simulink library "ee_lib_for_power_systems"  (R2025b)
settings: Solver=VariableStepAuto  StartTime=0.0  StopTime=10.0  RelTol=1e-3
undefined symbols (not defined in the file or in its folder): Alt_PolePair_Count, C3m, DiscIntNeverNeededParam, Enum1to12, L3m, Total_SM_Inertia, Ts
libraries: fl_lib x291, nesl_utility x219, ee_lib x139, custom_lib x12, $bdroot x10, ee_sl_lib x3, eeTransforms x2, eeGeneralControl x1, simulink x1
(part 2 of 11)

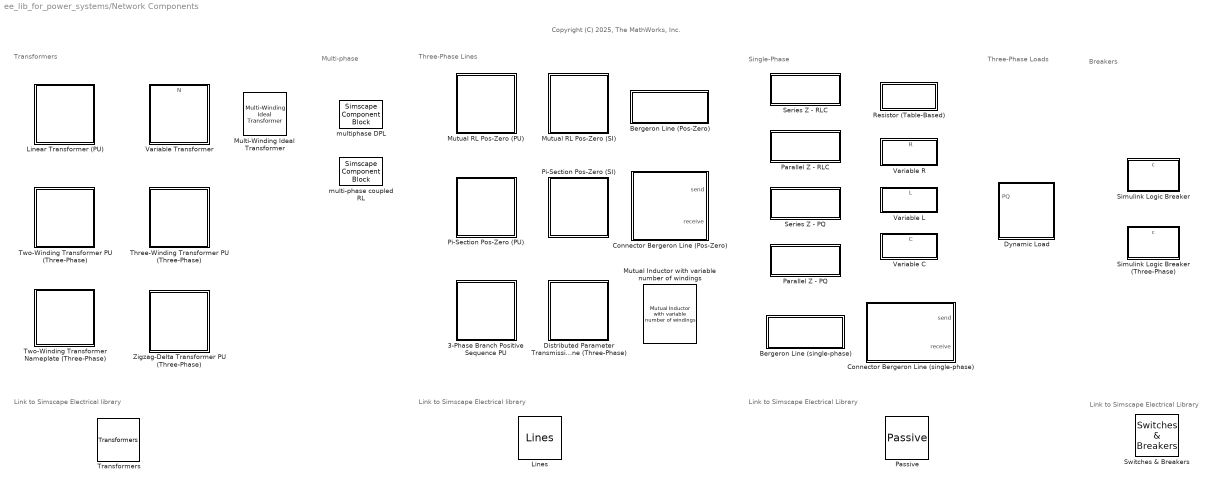
== system ee_lib_for_power_systems/Network Components ==
  note: Multi-phase
  note: Link to Simscape Electrical Library
  note: Link to Simscape Electrical Library
  note: Link to Simscape Electrical library
  note: Link to Simscape Electrical library
  note: Three-Phase Loads
  note: Breakers
  note: Single-Phase
  note: Three-Phase Lines
  note: Transformers
  note: Copyright (C) 2025, The MathWorks, Inc.
  " Three-Winding Transformer PU (Three-Phase)" : Subsystem  mask{conn1=Yg, conn2=delta1, conn3=Y, core=fivelimb, Pnom_Fnom=[250e6 60], V1_R1_L1=[300000 0.01 0.05], V2_R2_L2=[50000 0.01 0.05], V3_R3_L3=[100000 0.01 0.05], Rm_Lm=[500 500], run_time=on}
      mask prompts: conn1 "Winding 1 Connection"; conn2 "Winding 2 Connection"; conn3 "Winding 3 Connection"; Pnom_Fnom "Nominal values [Pnom(VA) Fnom(Hz)]"; V1_R1_L1 "Winding 1 [V1(Vrms) R1(pu) L1(pu)]"; V2_R2_L2 "Winding 2 [V2(Vrms) R2(pu) L2(pu)]"; V3_R3_L3 "Winding 3 [V3(Vrms) R3(pu) L3(pu)]"; Rm_Lm "Magnetization [Rm (pu) Lm(pu)]"; run_time "Set parameters to run-time (model does not recompile if parameters change value)"
      mask init:
      | maskObj = Simulink.Mask.get(gcbh);
      | workspaceObj = maskObj.getWorkspace();
      | 
      | Pnom_Fnom = workspaceObj.get('Pnom_Fnom');
      | V1_R1_L1 = workspaceObj.get('V1_R1_L1');
      | V2_R2_L2 = workspaceObj.get('V2_R2_L2');
      | V3_R3_L3 = workspaceObj.get('V3_R3_L3');
      | Rm_Lm = workspaceObj.get('Rm_Lm');
      | 
      | R1base = V1_R1_L1(1)^2/Pnom_Fnom(1);
      | X1base = V1_R1_L1(1)^2/Pnom_Fnom(1)/(2*pi*Pnom_Fnom(2));
      | 
      | R2base = V2_R2_L2(1)^2/Pnom_Fnom(1);
      | X2base = V2_R2_L2(1)^2/Pnom_Fnom(1)/(2*pi*Pnom_Fnom(2));
      | 
      | R3base = V3_R3_L3(1)^2/Pnom_Fnom(1);
      | X3base = V3_R3_L3(1)^2/Pnom_Fnom(1)/(2*pi*Pnom_Fnom(2));
      | 
      | V1 = V1_R1_L1(1);
      | V2 = V2_R2_L2(1);
      | V3 = V3_R3_L3(1);
      | 
      | R1_pu = V1_R1_L1(2);
      | L1_pu = V1_R1_L1(3);
      | R2_pu = V2_R2_L2(2);
      | L2_pu = V2_R2_L2(3);
      | R3_pu = V3_R3_L3(2);
      | L3_pu = V3_R3_L3(3);
      | Rm_pu = Rm_Lm(1);
      | Lm_pu = Rm_Lm(2);
      |     
      | path_Transformer = [gcb,'/Transformer'];
      | 
      | conn1 = get_param(gcb,'conn1');
      | conn2 = get_param(gcb,'conn2');
      | conn3 = get_param(gcb,'conn3');
      | core  = get_param(gcb,'core');
      | 
      | set_param(path_Transformer,'Winding1Connection',['ee.enum.windingconnection.',conn1])
      | set_param(path_Transformer,'Winding2Connection',['ee.enum.windingconnection.',conn2])
      | set_param(path_Transformer,'Winding3Connection',['ee.enum.windingconnection.',conn3])
      | set_param(path_Transformer,'CoreType',['ee.enum.coretype.',core])
      mask TypeOptions conn1: Y | Yg | delta1 | delta11
      mask TypeOptions conn2: Y | Yg | delta1 | delta11
      mask TypeOptions conn3: Y | Yg | delta1 | delta11
      mask TypeOptions core: threelimb | fivelimb
      mask Callback run_time:
      | run_time = get_param(gcb,'run_time');
      | 
      | path_Transformer = [gcb,'/Transformer'];
      | 
      | switch run_time
      |     case 'off'
      |         pcr = 'compiletime';
      |     case 'on'
      |         pcr = 'runtime';
      | end
      | 
      | set_param(path_Transformer,'VRated1_conf',pcr)
      | set_param(path_Transformer,'VRated2_conf',pcr)
      | set_param(path_Transformer,'VRated3_conf',pcr)
      | set_param(path_Transformer,'SRated_conf',pcr)
      | set_param(path_Transformer,'FRated_conf',pcr)
      | set_param(path_Transformer,'pu_Rm_conf',pcr)
      | set_param(path_Transformer,'pu_Xm_conf',pcr)
      | set_param(path_Transformer,'pu_Rw1_conf',pcr)
      | set_param(path_Transformer,'pu_Xl1_conf',pcr)
      | set_param(path_Transformer,'pu_Rw2_conf',pcr)
      | set_param(path_Transformer,'pu_Xl2_conf',pcr)
      | set_param(path_Transformer,'pu_Rw3_conf',pcr)
      | set_param(path_Transformer,'pu_Xl3_conf',pcr)
      mask DialogControl DescGroupVar Prompt: Three-Winding Transformer
      mask DialogControl Container4 Prompt: Configure
      mask DialogControl Container5 Prompt: Parameters
      mask Display:
      | color('lightblue')
      | 
      | ph = linspace(0,2*pi,200);
      | 
      | plot(0.2*sin(ph)+0.4,0.2*cos(ph)+0.5)
      | plot(0.2*sin(ph)+0.6,0.2*cos(ph)+0.62)
      | plot(0.2*sin(ph)+0.6,0.2*cos(ph)+0.38)
      | 
      | plot([0.1 0.2],[0.5 0.5])
      | plot([0.75 0.9],[0.25 0.25])
      | plot([0.75 0.9],[0.75 0.75])
      | plot(0,0)
      | plot(1,1)
      | 
      | conn1 = get_param(gcbh,'conn1');
      | cc1 = conn1;
      | if strcmp(conn1,'delta1')
      |     cc1 = 'D1';
      | elseif strcmp(conn1,'delta11')
      |     cc1 = 'D11';
      | end
      | 
      | text(0.2,0.25,cc1)
      | 
      | conn2 = get_param(gcbh,'conn2');
      | cc2 = conn2;
      | if strcmp(conn2,'delta1')
      |     cc2 = 'D1';
      | elseif strcmp(conn2,'delta11')
      |     cc2 = 'D11';
      | end
      | 
      | text(0.7,0.9,cc2)
      | 
      | conn3 = get_param(gcbh,'conn3');
      | cc3 = conn3;
      | if strcmp(conn3,'delta1')
      |     cc3 = 'D1';
      | elseif strcmp(conn3,'delta11')
      |     cc3 = 'D11';
      | end
      | 
      | text(0.7,0.15 ,cc3)
  3-Phase Branch Positive Sequence PU : Subsystem  mask{Pnom_Fnom=[5e6 60], Tx=off, Vnom1=5e3, Vnom2=2e3, R=0.1, X=0.1, B=0.1, run_time=on}
      mask prompts: Pnom_Fnom "Nominal values [Pnom(VA) Fnom(Hz)]"; Tx "Include Ideal Transformer"; Vnom1 "Port 1 Nominal Voltage (Vrms)"; Vnom2 "Nominal Voltage (Vrms)"; R "Series Resistance R (pu)"; X "Series Reactance X (pu)"; B "Shunt Line Charging Susceptance B (pu)"; run_time "Set parameters to run-time (avoid recompile if parameters change value)"
      mask init:
      | maskObj = Simulink.Mask.get(gcbh);
      | workspaceObj = maskObj.getWorkspace();
      | 
      | % Pnom_Vnom = workspaceObj.get('Pnom_Fnom');
      | % Vnom1 = workspaceObj.get('Vnom1');
      | % Vnom2 = workspaceObj.get('Vnom2');
      | 
      | R = workspaceObj.get('R');
      | X = workspaceObj.get('X');
      | B = workspaceObj.get('B');
      | 
      | path_RLC   = [gcb,'/RLC'];
      | path_C1    = [gcb,'/C1'];
      | path_C2    = [gcb,'/C2'];
      | path_gnd1  = [gcb,'/gnd1'];
      | path_gnd2  = [gcb,'/gnd2'];
      | 
      | 
      | if R>0 && X>0 % set branch to RL
      | 
      |     set_param(path_RLC,'component_structure','ee.enum.rlc.structure.SeriesRL');
      | 
      | elseif R>0 && X<0 % set branch to RC
      | 
      |     set_param(path_RLC,'component_structure','ee.enum.rlc.structure.SeriesRC');
      | 
      | elseif R==0 && X>0 % set branch to L
      | 
      |     set_param(path_RLC,'component_structure','ee.enum.rlc.structure.L');
      | 
      | elseif R==0 && X<0 % set branch to C
      | 
      |     set_param(path_RLC,'component_structure','ee.enum.rlc.structure.C');
      | 
      | end
      | 
      | if B>0 
      | 
      |     set_param(path_C1,'Commented','off')
      |     set_param(path_C2,'Commented','off')
      |     set_param(path_gnd1,'Commented','off')
      |     set_param(path_gnd2,'Commented','off')
      | 
      | elseif B==0 % comment out shunt
      | 
      |     set_param(path_C1,'Commented','on');
      |     set_param(path_C2,'Commented','on');
      |     set_param(path_gnd1,'Commented','on')
      |     set_param(path_gnd2,'Commented','on')
      |     
      | end
      mask Callback Tx:
      | Tx = get_param(gcb,'Tx');
      | 
      | path_Tx  = [gcb,'/Tx'];
      | 
      | mp  ={
      |     'Nominal values [Pnom(VA) Fnom(Hz)]'
      |     'Include Ideal Transformer'
      |     'Port 1 Nominal Voltage (Vrms)'
      |     'Nominal Voltage (Vrms)'
      |     'Series Resistance R (pu)'
      |     'Series Reactance X (pu)'
      |     'Shunt Line Charging Susceptance B (pu)'
      |     'Set parameters to run-time (avoid recompile if parameters change value)'
      |     };
      | 
      | set_param(gcb,'MaskPrompts',mp)
      | 
      | switch Tx
      | 
      |     case 'off'
      | 
      |         set_param(gcb,'MaskVisibilityString','on,on,off,on,on,on,on,on')
      |         set_param(path_Tx,'Commented','through')
      | 
      |     case 'on'
      | 
      |         set_param(gcb,'MaskVisibilityString','on,on,on,on,on,on,on,on')
      |         mp{4} = 'Port 2 Nominal Voltage (Vrms)';
      |         set_param(path_Tx,'Commented','off')
      | 
      | end
      | 
      | set_param(gcb,'MaskPrompts',mp)
      mask Callback run_time:
      | run_time = get_param(gcb,'run_time');
      | 
      | path_RLC   = [gcb,'/RLC'];
      | path_C1    = [gcb,'/C1'];
      | path_C2    = [gcb,'/C2'];
      | path_TtxA  = [gcb,'/Tx/TtxA'];
      | path_TtxB  = [gcb,'/Tx/TtxB'];
      | path_TtxC  = [gcb,'/Tx/TtxC'];
      | 
      | switch run_time
      |     case 'off'
      |         pcr = 'compiletime';
      |     case 'on'
      |         pcr = 'runtime';
      | end
      | 
      | set_param(path_RLC,'R_conf',pcr)
      | set_param(path_RLC,'L_conf',pcr)
      | set_param(path_RLC,'C_conf',pcr)
      | set_param(path_C1,'C_conf',pcr)
      | set_param(path_C2,'C_conf',pcr)
      | set_param(path_C1,'L_conf',pcr)
      | set_param(path_C2,'L_conf',pcr)
      | set_param(path_TtxA,'n_conf',pcr)
      | set_param(path_TtxB,'n_conf',pcr)
      | set_param(path_TtxC,'n_conf',pcr)
      mask DialogControl DescGroupVar Prompt: 3-Phase Branch Positive Sequence PU
      mask DialogControl DescTextVar Prompt: This branch is parameterized from positive sequence data in R, X, B format. Where R is series resistance, X is series reactance and B is line charging susceptance. For series inductance X is positive. For series capacitance, X is negative. When included, the ideal transformer is connected at port 1, and the RLC per-unit values are referred to the voltage at port 2.
      mask DialogControl Container5 Prompt: Parameters
      mask Display: disp('Three-Phase\nBranch\nPos-Seq (PU)')
  Bergeron Line (Pos-Zero) : Subsystem  mask{length=100, R1_L1_C1=[0.01 0.001 1e-8], R0_L0_C0=[0.4 0.004 8e-9], Ts=5e-5}
      mask prompts: length "Length (km)"; R1_L1_C1 "Positive sequence [R1 (Ohm/km)  L1 (H/km)  C1 (F/km)]"; R0_L0_C0 "Zero sequence [R0 (Ohm/km)  L0 (H/km)  C0 (F/km)]"; Ts "Sample time (s)"
      mask init:
      | maskObj = Simulink.Mask.get(gcb);
      | workspaceObj = maskObj.getWorkspace();
      | 
      | length   = workspaceObj.get('length');
      | R1_L1_C1 = workspaceObj.get('R1_L1_C1');
      | R0_L0_C0 = workspaceObj.get('R0_L0_C0');
      | 
      | R1 = R1_L1_C1(1);
      | L1 = R1_L1_C1(2);
      | C1 = R1_L1_C1(3);
      | 
      | R0 = R0_L0_C0(1);
      | L0 = R0_L0_C0(2);
      | C0 = R0_L0_C0(3);
      | 
      | 
      | Rp = (2*R1 + R0)/3;
      | Rm = (R0 - R1)/3;
      | 
      | Lp = (2*L1 + L0)/3;
      | Lm = (L0 - L1)/3;
      | 
      | Cp = (2*C1 + C0)/3;
      | Cm = (C0 - C1)/3;
      | 
      | 
      | % Clarke Transform for alpha-beta-zero to ABC
      | 
      | T_clarke = [ 2/sqrt(6)  0         1/sqrt(3)
      |             -1/sqrt(6)  1/sqrt(2) 1/sqrt(3)
      |             -1/sqrt(6) -1/sqrt(2) 1/sqrt(3)];
      | 
      | R_alpha = length*(Rp - Rm);
      | L_alpha = length*(Lp - Lm);
      | C_alpha = length*(Cp - Cm);
      | 
      | R_beta = length*(Rp - Rm);
      | L_beta = length*(Lp - Lm);
      | C_beta = length*(Cp - Cm);
      | 
      | R_zero = length*(Rp + 2*Rm);
      | L_zero = length*(Lp + 2*Lm);
      | C_zero = length*(Cp + 2*Cm);
      | 
      | Zlc_alpha = sqrt(L_alpha/C_alpha);
      | Zlc_beta  = sqrt(L_beta/C_beta);
      | Zlc_zero  = sqrt(L_zero/C_zero);
      | 
      | tau_alpha = sqrt(L_alpha*C_alpha);
      | tau_beta  = sqrt(L_beta*C_beta);
      | tau_zero  = sqrt(L_zero*C_zero);
      | 
      | h_alpha = (Zlc_alpha - R_alpha/4)/(Zlc_alpha + R_alpha/4);
      | h_beta  = (Zlc_beta - R_beta/4)/(Zlc_beta + R_beta/4);
      | h_zero  = (Zlc_zero - R_zero/4)/(Zlc_zero + R_zero/4);
      | 
      | Zc = T_clarke*diag([Zlc_alpha + R_alpha/4 Zlc_beta + R_beta/4 Zlc_zero + R_zero/4])*T_clarke';
      mask DialogControl DescGroupVar Prompt: Bergeron Line (Pos-Zero)
      mask DialogControl DescTextVar Prompt: Three-phase Bergeron line modeled in the Clarke domain and parameterized with positive- and zero-sequence values.
      mask Display: disp('Bergeron Line\n (Pos-Zero)')
  Bergeron Line (single-phase) : Subsystem  mask{length=100, freq=60, R1_L1_C1=[0.01 0.001 1e-8], Ts=5e-5}
      mask prompts: length "Length (km)"; freq "Frequency (Hz)"; R1_L1_C1 "[R (Ohm/km)  L (H/km)  C (F/km)]"; Ts "Sample time (s)"
      mask init:
      | maskObj = Simulink.Mask.get(gcb);
      | workspaceObj = maskObj.getWorkspace();
      | 
      | length   = workspaceObj.get('length');
      | R1_L1_C1 = workspaceObj.get('R1_L1_C1');
      | 
      | R1 = R1_L1_C1(1);
      | L1 = R1_L1_C1(2);
      | C1 = R1_L1_C1(3);
      | 
      | R = length*R1;
      | L = length*L1;
      | C = length*C1;
      | 
      | Zlc = sqrt(L/C);
      | 
      | tau = sqrt(L*C);
      | 
      | h = (Zlc - R/4)/(Zlc + R/4);
      | 
      | Zc = Zlc + R/4;
      mask DialogControl DescGroupVar Prompt: Bergeron Line (single-phase)
      mask DialogControl DescTextVar Prompt: Single-phase Bergeron line.
      mask Display: disp('Bergeron Line\n (single-phase)')
  Connector Bergeron Line (Pos-Zero) : Subsystem  mask{length=100, R1_L1_C1=[0.01 0.001 1e-8], R0_L0_C0=[0.4 0.004 8e-9], Ts=5e-5}
      mask prompts: length "Length (km)"; R1_L1_C1 "Positive sequence [R1 (Ohm/km) L1 (H/km) C1 (F/km)]"; R0_L0_C0 "Negative sequence [R0 (Ohm/km) L0 (H/km) C0 (F/km)]"; Ts "Sample time (s)"
      mask init: same as ee_lib_for_power_systems/Network Components/Bergeron Line (Pos-Zero)
      settings: PortSchema="{\"entries\":[{\"content\":{\"sides\":[{\"content\":{\"connectorIds\":[\"Out1\",\"In1\"]},\"type\":\"ConnectorPlacement.EquallySpacedRectSide\",\"uuid\":\"9f5ca3d6-f6a4-41cb-8fcc-925cc537c09c\"},{\"content\":{\"connectorIds\":[\"RConn1\"],\"side\":\"RIGHT\"},\"type\":\"ConnectorPlacement.EquallySpacedRectSide\",\"uuid\":\"b3e1c921-5439-47a2-9320-548c916c4381\"},{\"content\":{\"side\":\"TOP\"},\"type\":\"ConnectorPlacement.EquallySpacedRectSide\",\"uuid\":\"4107acad-41a5-46f1-8256-3fe80bf4ab25\"}]},\"type\":\"ConnectorPlacement.EquallySpacedConnector\",\"uuid\":\"49d42ba1-f406-47e2-8b36-5894a8644831\"}],\"packageUris\":[\"http://schema.mathworks.com/mf0/Connector/R2020b\"],\"version\":\"1.0\"}"
      mask DialogControl DescGroupVar Prompt: Connector Bergeron Line
      mask DialogControl DescTextVar Prompt: Three-phase Bergeron line modeled in Clarke domain and parameterized with positive- and negative-sequence values. Copy this block and connect the two blocks together, using external unit delays equal to sample time, to support model partitioning.
      mask Display: disp('Connector\nBergeron Line\n(Pos-Zero)')
  Connector Bergeron Line (single-phase) : Subsystem  mask{length=100, R1_L1_C1=[0.01 0.001 1e-8], Ts=5e-5}
      mask prompts: length "Length (km)"; R1_L1_C1 "[R (Ohm/km) L (H/km) C (F/km)]"; Ts "Sample time (s)"
      mask init: same as ee_lib_for_power_systems/Network Components/Bergeron Line (single-phase)
      settings: PortSchema="{\"entries\":[{\"content\":{\"sides\":[{\"content\":{\"connectorIds\":[\"LConn1\"]},\"type\":\"ConnectorPlacement.EquallySpacedRectSide\",\"uuid\":\"cf1dac5e-4044-4306-9dbf-59a41e541168\"},{\"content\":{\"connectorIds\":[\"Out1\",\"In1\"],\"side\":\"RIGHT\"},\"type\":\"ConnectorPlacement.EquallySpacedRectSide\",\"uuid\":\"dfadc406-ca2c-4f51-8800-efbfc751a344\"},{\"content\":{\"side\":\"TOP\"},\"type\":\"ConnectorPlacement.EquallySpacedRectSide\",\"uuid\":\"d7ba0d90-105a-4c49-afe3-68814fc61bad\"}]},\"type\":\"ConnectorPlacement.EquallySpacedConnector\",\"uuid\":\"7281a799-d4b8-497c-b81f-a7465f7a7d6a\"}],\"packageUris\":[\"http://schema.mathworks.com/mf0/Connector/R2020b\"],\"version\":\"1.0\"}"
      mask DialogControl DescGroupVar Prompt: Connector Bergeron Line (single-phase)
      mask DialogControl DescTextVar Prompt: Single-phase Bergeron line. Copy this block and connect the two blocks together, using external unit delays equal to sample time, to support model partitioning.
      mask Display: disp('Connector\nBergeron Line\n(single-phase)')
  "Distributed Parameter Transmission Line (Three-Phase) " : Subsystem  mask{length=1, Fnom=60, R1_L1_C1=[1e-2 1e-3 15e-9], R0_L0_C0=[0.2 5e-3 5e-9], run_time=off}
      mask prompts: length "Line Length (km)"; Fnom "Frequency used for rlcg specification (Hz)"; R1_L1_C1 "Positive Sequence [R1(Ohm/km) L1(H/km) C1(F/km)]"; R0_L0_C0 "Zero Sequence [R0(Ohm/km) L0(H/km) C0(F/km)]"; run_time "Set parameters to run-time (model does not recompile if parameters change value)"
      mask init:
      | maskObj = Simulink.Mask.get(gcbh);
      | workspaceObj = maskObj.getWorkspace();
      | 
      | length = workspaceObj.get('length');
      | Fnom = workspaceObj.get('Fnom');
      | R1_L1_C1 = workspaceObj.get('R1_L1_C1');
      | R0_L0_C0 = workspaceObj.get('R0_L0_C0');
      | 
      | R1 = R1_L1_C1(1);
      | L1 = R1_L1_C1(2);
      | C1 = R1_L1_C1(3);
      | 
      | R0 = R0_L0_C0(1);
      | L0 = R0_L0_C0(2);
      | C0 = R0_L0_C0(3);
      mask Callback run_time:
      | run_time = get_param(gcb,'run_time');
      | 
      | path_line   = [gcb,'/distributed_parameter_line'];
      | 
      | switch run_time
      |     case 'off'
      |         pcr = 'compiletime';
      |     case 'on'
      |         pcr = 'runtime';
      | end
      | 
      | set_param(path_line,'freq_conf',pcr)
      | set_param(path_line,'length_conf',pcr)
      | set_param(path_line,'R1_conf',pcr)
      | set_param(path_line,'L1_conf',pcr)
      | set_param(path_line,'C1_conf',pcr)
      | set_param(path_line,'R0_conf',pcr)
      | set_param(path_line,'L0_conf',pcr)
      | set_param(path_line,'C0_conf',pcr)
      mask DialogControl DescGroupVar Prompt: Distributed Parameter Line (Three-Phase)
      mask DialogControl DescTextVar Prompt: Define distributed parameter line with positive and zero sequence values
      mask DialogControl Container5 Prompt: Parameters
      mask Display: disp('Distributed\nParameter\nLine (3ph)')
  Dynamic Load : Subsystem  mask{Fnom=60, Vnom=24e3, run_time=off}
      mask prompts: Fnom "Nominal Frequency (Hz)"; Vnom "Nominal Voltage (Vrms)"; run_time "Set parameters to run-time (model does not recompile if parameters change value)"
      mask Callback run_time:
      | run_time = get_param(gcb,'run_time');
      | 
      | path_pq = [gcb,'/pq_load'];
      | 
      | switch run_time
      |     case 'off'
      |         pcr = 'compiletime';
      |     case 'on'
      |         pcr = 'runtime';
      | end
      | 
      | set_param(path_pq,'Vline_rms_ini_conf',pcr)
      | set_param(path_pq,'FRated_conf',pcr)
      mask DialogControl DescGroupVar Prompt: Dynamic PQ Load
      mask DialogControl DescTextVar Prompt: Thee-phase balanced PQ load with external inputs.
      mask Display: disp('PQ\nLoad')
  Linear Transformer (PU) : Subsystem  mask{Pnom_Fnom=[250e6 60], V1_V2=[500000 250000], R_X=[0.01 0.08], Rm_Xm=[500 500], run_time=off}
      mask prompts: Pnom_Fnom "Nominal values [Pnom(VA) Fnom(Hz)]"; V1_V2 "Primary and Secondary Voltages[V1(Vrms) V2(Vrms)]"; R_X "Winding Resistance and Leakage Reactance [R(pu) X(pu)]"; Rm_Xm "Magnetization [Rm(pu) Xm(pu)]"; run_time "Set parameters to run-time (model does not recompile if parameters change value)"
      mask init:
      | maskObj = Simulink.Mask.get(gcbh);
      | workspaceObj = maskObj.getWorkspace();
      | 
      | Pnom_Fnom = workspaceObj.get('Pnom_Fnom');
      | V1_V2 = workspaceObj.get('V1_V2');
      | R_X = workspaceObj.get('R_X');
      | Rm_Xm = workspaceObj.get('Rm_Xm');
      | 
      | V1 = V1_V2(1);
      | V2 = V1_V2(2);
      | 
      | R1_pu = R_X(1)/2;
      | L1_pu = R_X(2)/2;
      | R2_pu = R_X(1)/2;
      | L2_pu = R_X(2)/2;
      | Rm_pu = Rm_Xm(1);
      | Lm_pu = Rm_Xm(2);
      | 
      | R1base = V1^2/Pnom_Fnom(1);
      | X1base = V1^2/Pnom_Fnom(1)/(2*pi*Pnom_Fnom(2));
      | 
      | R2base = V2^2/Pnom_Fnom(1);
      | X2base = V2^2/Pnom_Fnom(1)/(2*pi*Pnom_Fnom(2));
      | 
      | R1_si = R1_pu*R1base;
      | L1_si = L1_pu*X1base;
      | R2_si = R2_pu*R2base;
      | L2_si = L2_pu*X2base;
      | Rm_si = Rm_pu*R1base;
      | Lm_si = Lm_pu*X1base;
      mask Callback run_time:
      | run_time = get_param(gcb,'run_time');
      | 
      | path_R1 = [gcb,'/R1'];
      | path_R2 = [gcb,'/R2'];
      | path_Rm = [gcb,'/Rm'];
      | path_L1 = [gcb,'/L1'];
      | path_L2 = [gcb,'/L2'];
      | path_Lm = [gcb,'/Lm'];
      | 
      | switch run_time
      |     case 'off'
      |         pcr = 'compiletime';
      |     case 'on'
      |         pcr = 'runtime';
      | end
      | 
      | set_param(path_R1,'R_conf',pcr)
      | set_param(path_R2,'R_conf',pcr)
      | set_param(path_Rm,'R_conf',pcr)
      | set_param(path_L1,'l_conf',pcr)
      | set_param(path_L2,'l_conf',pcr)
      | set_param(path_Lm,'l_conf',pcr)
      mask DialogControl DescGroupVar Prompt: Ideal Transformer
      mask DialogControl DescTextVar Prompt: This block represents an ideal transformer.
      mask DialogControl Container5 Prompt: Parameters
      mask Display:
      | color('blue')
      | 
      | phl = linspace(0,-pi,200);
      | phr = linspace(0,pi,200);
      | 
      | r1 = 0.07;
      | b1 = 0.3;
      | 
      | plot(r1*sin(phl)+0.44,r1*cos(phl)+b1)
      | plot(r1*sin(phl)+0.44,r1*cos(phl)+b1+2*r1)
      | plot(r1*sin(phl)+0.44,r1*cos(phl)+b1+4*r1)
      | plot(r1*sin(phl)+0.44,r1*cos(phl)+b1+6*r1)
      | 
      | plot(r1*sin(phr)+0.56,r1*cos(phr)+b1)
      | plot(r1*sin(phr)+0.56,r1*cos(phr)+b1+2*r1)
      | plot(r1*sin(phr)+0.56,r1*cos(phr)+b1+4*r1)
      | plot(r1*sin(phr)+0.56,r1*cos(phr)+b1+6*r1)
      | 
      | 
      | %plot(0.05*sin(phr)+0.6,0.05*cos(phr)+0.5)
      | 
      | plot(0,0)
      | plot(1,1)
      | plot([0.2 0.44],[0.85 0.85])
      | plot([0.56 0.8],[0.85 0.85])
      | plot([0.2 0.44],[0.15 0.15])
      | plot([0.56 0.8],[0.15 0.15])
      | plot([0.56 0.56],[0.85 0.79])
      | plot([0.44 0.44],[0.85 0.79])
      | plot([0.56 0.56],[0.15 0.23])
      | plot([0.44 0.44],[0.15 0.23])
  Lines : ee_lib/Passive/Lines  Tag=simscape_sublibrary  SystemSampleTime=-1  VariantActivationTime=update diagram  EmptyChoice=off  PassThrough=off  PassThroughVariantControl=(default)  EmptyChoiceVariantControl=(default)  AllowFlexibleInterface=on  Latency=0  AutoFrameSizeCalculation=off
  Multi-Winding Ideal Transformer : custom_lib/Multi-Winding Ideal Transformer  npw=4  nsw=4  nVp=[1, 1, 1, 1] V  nVs=[1, 1, 1, 1] V
      settings: InternalSimscapePortConfiguration="{\"Bottom\":[],\"Left\":[{\"id\":\"pp\",\"label\":\"pp\",\"type\":\"foundation.electrical.electrical\"},{\"id\":\"np\",\"label\":\"np\",\"type\":\"foundation.electrical.electrical\"}],\"Right\":[{\"id\":\"ps\",\"label\":\"ps\",\"type\":\"foundation.electrical.electrical\"},{\"id\":\"ns\",\"label\":\"ns\",\"type\":\"foundation.electrical.electrical\"}],\"Top\":[]}"
  Mutual Inductor with variable number of windings : custom_lib/Mutual Inductor with variable number of windings  N=Enum1to12.three  length=1 km  L=[1, .1, .1; .1, 1, .1; .1, .1, 1] H/km  R=[1, .1, .1; .1, 1, .1; .1, .1, 1] Ohm/km
      settings: InternalSimscapePortConfiguration="{\"Bottom\":[],\"Left\":[{\"id\":\"p1\",\"label\":\"p1\",\"type\":\"foundation.electrical.electrical\"},{\"id\":\"p2\",\"label\":\"p2\",\"type\":\"foundation.electrical.electrical\"},{\"id\":\"p3\",\"label\":\"p3\",\"type\":\"foundation.electrical.electrical\"}],\"Right\":[{\"id\":\"n1\",\"label\":\"n1\",\"type\":\"foundation.electrical.electrical\"},{\"id\":\"n2\",\"label\":\"n2\",\"type\":\"foundation.electrical.electrical\"},{\"id\":\"n3\",\"label\":\"n3\",\"type\":\"foundation.electrical.electrical\"}],\"Top\":[]}"
  Mutual RL Pos-Zero (PU) : Subsystem  mask{Pnom_Vnom_Fnom=[5e6 5e3 60], R1_L1=[2 0.05], R0_L0=[4 0.1], run_time=off}
      mask prompts: Pnom_Vnom_Fnom "Nominal values [Pnom(VA) Vnom(Vrms) Fnom(Hz)]"; R1_L1 "Positive Sequence [R1(pu) L1(pu)]"; R0_L0 "Zero Sequence [R0(pu) L0(pu)]"; run_time "Set parameters to run-time (model does not recompile if parameters change value)"
      mask init:
      | maskObj = Simulink.Mask.get(gcbh);
      | workspaceObj = maskObj.getWorkspace();
      | 
      | Pnom_Vnom_Fnom = workspaceObj.get('Pnom_Vnom_Fnom');
      | R1_L1 = workspaceObj.get('R1_L1');
      | R0_L0 = workspaceObj.get('R0_L0');
      | 
      | Pnom = Pnom_Vnom_Fnom(1);
      | Vnom = Pnom_Vnom_Fnom(2);
      | Fnom = Pnom_Vnom_Fnom(3);
      | 
      | Rbase = Vnom^2/Pnom(1);
      | Lbase = Rbase/(2*pi*Fnom);
      | 
      | R1_si = R1_L1(1)*Rbase;
      | L1_si = R1_L1(2)*Lbase;
      | R0_si = R0_L0(1)*Rbase;
      | L0_si = R0_L0(2)*Lbase;
      | 
      | Rs_si = (2*R1_si + R0_si)/3;
      | Rm_si = (R0_si - R1_si)/3;
      | 
      | Ls_si = (2*L1_si + L0_si)/3;
      | Lm_si = (L0_si - L1_si)/3;
      mask Callback run_time:
      | run_time = get_param(gcb,'run_time');
      | 
      | path_coupled = [gcb,'/Coupled'];
      | 
      | switch run_time
      |     case 'off'
      |         pcr = 'compiletime';
      |     case 'on'
      |         pcr = 'runtime';
      | end
      | 
      | set_param(path_coupled,'R_conf',pcr)
      | set_param(path_coupled,'Rm_conf',pcr)
      | set_param(path_coupled,'L_conf',pcr)
      | set_param(path_coupled,'Lm_conf',pcr)
      mask DialogControl DescGroupVar Prompt: Mutual RL Pos-Zero
      mask DialogControl DescTextVar Prompt: Define mutual RL with positive and zero sequence values
      mask DialogControl Container5 Prompt: Parameters
      mask Display: disp('Mutual RL\n Pos-Zero\n(PU)')
  Mutual RL Pos-Zero (SI) : Subsystem  mask{R1_L1=[2 0.05], R0_L0=[4 0.1], run_time=off}
      mask prompts: R1_L1 "Positive Sequence [R1(Ohm) L1(H)]"; R0_L0 "Zero Sequence [R0(Ohm) L0(H)]"; run_time "Set parameters to run-time (model does not recompile if parameters change value)"
      mask init:
      | maskObj = Simulink.Mask.get(gcbh);
      | workspaceObj = maskObj.getWorkspace();
      | 
      | R1_L1 = workspaceObj.get('R1_L1');
      | R0_L0 = workspaceObj.get('R0_L0');
      | 
      | R1_si = R1_L1(1);
      | L1_si = R1_L1(2);
      | R0_si = R0_L0(1);
      | L0_si = R0_L0(2);
      | 
      | Rs_si = (2*R1_si + R0_si)/3;
      | Rm_si = (R0_si - R1_si)/3;
      | 
      | Ls_si = (2*L1_si + L0_si)/3;
      | Lm_si = (L0_si - L1_si)/3;
      mask Callback run_time:
      | run_time = get_param(gcb,'run_time');
      | 
      | path_coupled = [gcb,'/Coupled'];
      | 
      | switch run_time
      |     case 'off'
      |         pcr = 'compiletime';
      |     case 'on'
      |         pcr = 'runtime';
      | end
      | 
      | set_param(path_coupled,'R_conf',pcr)
      | set_param(path_coupled,'Rm_conf',pcr)
      | set_param(path_coupled,'L_conf',pcr)
      | set_param(path_coupled,'Lm_conf',pcr)
      mask DialogControl DescGroupVar Prompt: Mutual RL Pos-Zero
      mask DialogControl DescTextVar Prompt: Define mutual RL with positive and zero sequence values
      mask DialogControl Container5 Prompt: Parameters
      mask Display: disp('Mutual RL\n Pos-Zero\n(SI)')
  Parallel Z - PQ : Subsystem  mask{Vnom_Fnom=[100 60], P=1e3, Q=1e3, run_time=off}
      mask prompts: Vnom_Fnom "Nominal Values [Vnom(Vrms) Fnom(Hz)]"; P "Active Power (W)"; Q "Reactive Power (Inductive +VAr, Capacitive -VAr)"; run_time "Set parameters to run-time (model does not recompile if parameters change value)"
      mask init:
      | maskObj = Simulink.Mask.get(gcbh);
      | workspaceObj = maskObj.getWorkspace();
      | 
      | Vnom_Fnom = workspaceObj.get('Vnom_Fnom');
      | P = workspaceObj.get('P');
      | Q = workspaceObj.get('Q');
      | 
      | path_R = [gcb,'/Resistor'];
      | path_L = [gcb,'/Inductor'];
      | path_C = [gcb,'/Capacitor'];
      | 
      | Vnom = Vnom_Fnom(1);
      | Fnom = Vnom_Fnom(2);
      | 
      | set_param(path_R,'Commented','off')
      | set_param(path_L,'Commented','off')
      | set_param(path_C,'Commented','off')
      | 
      | R = Vnom^2/P;
      | 
      | if P == 0
      |     set_param(path_R,'commented','on')
      | end
      | 
      | if Q > 0
      |     L = Vnom^2/abs(Q)/(2*pi*Fnom);
      |     set_param(path_C,'Commented','on')
      | elseif Q<0
      |     C = abs(Q)/Vnom^2/(2*pi*Fnom);
      |     set_param(path_L,'Commented','on')
      | elseif Q==0
      |     set_param(path_L,'Commented','on')
      |     set_param(path_C,'Commented','on')
      | end
      mask DialogControl DescGroupVar Prompt: Parallel Z - PQ
      mask DialogControl DescTextVar Prompt: Define parallel constant impedance through P and Q values
      mask Display: disp('Parallel Z - PQ')
  Parallel Z - RLC : Subsystem  mask{R=0.01, L=0.001, C=1e-5, run_time=off}
      mask prompts: R "Resistance (Ohm)"; L "Inductance (H)"; C "Capacitance (F)"; run_time "Set parameters to run-time (model does not recompile if parameters change value)"
      mask init:
      | maskObj = Simulink.Mask.get(gcbh);
      | workspaceObj = maskObj.getWorkspace();
      | 
      | R = workspaceObj.get('R');
      | L = workspaceObj.get('L');
      | C = workspaceObj.get('C');
      | 
      | 
      | hR = find_system(gcbh,'FollowLinks','on','FindAll','on','IncludeCommented','on','LookUnderMasks','on','Name','Resistor');
      | hL = find_system(gcbh,'FollowLinks','on','FindAll','on','IncludeCommented','on','LookUnderMasks','on','Name','Inductor');